simulink model "correlator_2bit_bee2_x2"  (Simulink 7.1)
settings: Solver=ode45  StartTime=0.0  StopTime=2048*5  RelTol=1e-3
StartFcn:
  | warning off Simulink:SL_LoadMdlParameterizedLink;
  | warning off Simulink:SL_OutputNotConnected;
  | warning off Simulink:SL_UsingDefaultMaxStepSize;
undefined symbols (not defined in the file or in its folder): BEE2_usr
libraries: xbsIndex_r4 x1264, cfa_library x320, xps_library x10, casper_library_misc x2
(part 1 of 15)
settings Model: Creator=rprimian  LastModifiedBy=Jonathan Weintroub
settings OptimizationCC: InitFltsAndDblsToZero=off

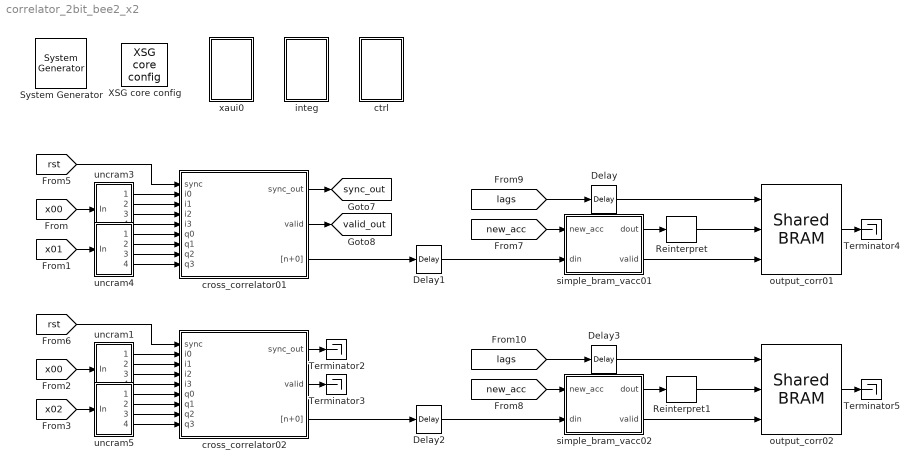
== system correlator_2bit_bee2_x2 ==
  " System Generator" : xbsIndex_r4/ System Generator  Tag=genX  SystemSampleTime=-1  xilinxfamily=virtex2p  part=xc2vp70  speed=-7  package=ff1704  synthesis_tool=XST  clock_wrapper=Clock Enables  directory=./correlator_2bit_bee2_x2/sysgen  testbench=off  simulink_period=1  sysclk_period=3.9063  dcm_input_clock_period=100  incr_netlist=off  trim_vbits=Everywhere in SubSystem  dbl_ovrd=According to Block Masks  core_generation=According to Block Masks  run_coregen=off  deprecated_control=off  eval_field=0  block_type=sysgen
      settings: block_version=11.3
  Delay : xbsIndex_r4/Delay  en=off  latency=2  dbl_ovrd=off  reg_retiming=off  block_type=delay
      settings: block_version=11.4
  Delay1 : xbsIndex_r4/Delay  en=off  latency=4  dbl_ovrd=off  reg_retiming=off  block_type=delay
      settings: block_version=11.4
  Delay2 : xbsIndex_r4/Delay  en=off  latency=4  dbl_ovrd=off  reg_retiming=off  block_type=delay
      settings: block_version=11.4
  Delay3 : xbsIndex_r4/Delay  en=off  latency=2  dbl_ovrd=off  reg_retiming=off  block_type=delay
      settings: block_version=11.4
  Reinterpret : xbsIndex_r4/Reinterpret  force_arith_type=on  arith_type=Unsigned  force_bin_pt=on  bin_pt=0  block_type=reinterpret
      settings: infoedit="Changes signal type without altering the binary representation.   You can changed the signal between signed and unsigned, and relocate the binary point.<br><br>Hardware notes: In hardware this block costs nothing.<br><br>Example:  Suppose the input is 6 bits wide, signed, with 2 fractional bits, and the output is forced to unsigned with 0 fractional bits.  Then an input of -2.0 (1110.00 in binary 2's complement) becomes an output of 56 (111000 in binary)."
  Reinterpret1 : xbsIndex_r4/Reinterpret  force_arith_type=on  arith_type=Unsigned  force_bin_pt=on  bin_pt=0  block_type=reinterpret
      settings: infoedit="Changes signal type without altering the binary representation.   You can changed the signal between signed and unsigned, and relocate the binary point.<br><br>Hardware notes: In hardware this block costs nothing.<br><br>Example:  Suppose the input is 6 bits wide, signed, with 2 fractional bits, and the output is forced to unsigned with 0 fractional bits.  Then an input of -2.0 (1110.00 in binary 2's complement) becomes an output of 56 (111000 in binary)."
  Terminator{2..5} (4 alike) : Terminator
  XSG core config : xps_library/XSG core config  Tag=xps:xsg  SystemSampleTime=-1  hw_sys=BEE2_usr  ibob_linux=off  clk_src=usr_clk2x  iBOB_clk_src=adc0_clk  BEE2_ctrl_clk_src=sys_clk  BEE2_usr_clk_src=usr_clk2x  ROACH_clk_src=adc0_clk  CORR_clk_src=sys_clk  gpio_clk_io_group=iBOB:sma  gpio_clk_bit_index=0  clk_rate=256  sample_period=1  synthesis_tool=XST  mpc_type=powerpc405
  cross_correlator01 : Subsystem  AncestorBlock=cfa_library/cross_correlator  mask(cross_correlator){total_lags=5, n_inputs=2, use_2bit=on, input_bit_width=8, input_bin_pt=7, specify_data_type=on, output_bit_width=12, output_bin_pt=0, quantization=Truncate, overflow=Wrap, specify_mult=off, mult_spec=Behavioral, specify_add=off, add_spec=Fabric, mult_delay=3, add_delay=2, single_output=on}
      mask prompts: total_lags "Total Lags Centered at Zero: (2^?)"; n_inputs "Number of Simultaneous Inputs: (2^?)"; use_2bit "Use special 2-bit binary encoding"; input_bit_width "Input Bit Width:"; input_bin_pt "Input Binary Point:"; specify_data_type "Specify output data type"; output_bit_width "Output Bit Width:"; output_bin_pt "Output Binary Point:"; quantization "Quantization Behavior:"; overflow "Overflow Behavior"; specify_mult "Specify multiplier use (behavioural HDL if not specified)"; mult_spec "Multiplier specification:"; specify_add "Specify adder use (behavioural HDL if not specified)"; add_spec "Adder specification:"; mult_delay "Mult Latency:"; add_delay "Add Latency:"; single_output "Provide single output stream"
      mask help: This time-domain cross correlator accepts two real time-series and outputs the cross-correlation function for the given set of lags. The output is accumulated so that for every two input samples there is exactly one output sample.
      mask init:
      | cross_correlator_init(gcb,...
      |     'total_lags', total_lags,...
      |     'n_inputs', n_inputs,...
      |     'use_2bit', use_2bit,...
      |     'input_bit_width', input_bit_width,...
      |     'input_bin_pt', input_bin_pt,...
      |     'specify_data_type', specify_data_type,...
      |     'output_bit_width', output_bit_width,...
      |     'output_bin_pt', output_bin_pt,...
      |     'quantization', quantization,...
      |     'overflow', overflow,...
      |     'specify_mult', specify_mult,...
      |     'mult_spec', mult_spec,...
      |     'specify_add', specify_add,...
      |     'add_spec', add_spec,...
      |     'mult_delay', mult_delay,...
      |     'add_delay', add_delay,...
      |     'single_output', single_output);
      settings: AttributesFormatString=|
      | 32 lags, Demux: 4, Outputs: 1
      | Special 2-bit binary encoding
      |  Mult: Behavioral, Add: Fabric
      mask StyleString: edit,edit,checkbox,edit,edit,checkbox,edit,edit,popup(Truncate|Round (unbiased: +/- Inf)|Round (unbiased: Even Values)),popup(Wrap|Saturate|Flag as error),checkbox,popup(Behavioral|Core|Embedded),checkbox,popup(Fabric|DSP48),edit,edit,checkbox
      mask CallbackString:
      | ||use_2bit = get_param(gcb,'use_2bit');
      | enables = get_param(gcb, 'MaskEnables');
      | if( strcmp(use_2bit,'on')),
      |     enables{4} = 'off';
      |     enables{5} = 'off';
      | else
      |     enables{4} = 'on';
      |     enables{5} = 'on';
      | end
      | set_param(gcb, 'MaskEnables', enables);
      | |||specify_data_type = get_param(gcb,'specify_data_type');
      | enables = get_param(gcb, 'MaskEnables');
      | if( strcmp(specify_data_type,'off')),
      |     enables{7} = 'off';
      |     enables{8} = 'off';
      |     enables{9} = 'off';
      |     enables{10} = 'off';
      | else
      |     enables{7} = 'on';
      |     enables{8} = 'on';
      |     enables{9} = 'on';
      |     enables{10} = 'on';
      | end
      | set_param(gcb, 'MaskEnables', enables);
      | |||||specify_mult = get_param(gcb,'specify_mult');
      | enables = get_param(gcb, 'MaskEnables');
      | if( strcmp(specify_mult,'off')),
      |     enables{12} = 'off';
      | else
      |     enables{12} = 'on';
      | end
      | set_param(gcb, 'MaskEnables', enables);
      | ||specify_add = get_param(gcb,'specify_add');
      | enables = get_param(gcb, 'MaskEnables');
      | if( strcmp(specify_add,'off')),
      |     enables{14} = 'off';
      | else
      |     enables{14} = 'on';
      | end
      | set_param(gcb, 'MaskEnables', enables);
      | ||||
      mask TabNameString: Basic,Basic,Input,Input,Input,Output,Output,Output,Output,Output,Implementation,Implementation,Implementation,Implementation,Implementation,Implementation,Basic
  cross_correlator02 : Subsystem  AncestorBlock=cfa_library/cross_correlator  mask(cross_correlator){total_lags=5, n_inputs=2, use_2bit=on, input_bit_width=8, input_bin_pt=7, specify_data_type=on, output_bit_width=12, output_bin_pt=0, quantization=Truncate, overflow=Wrap, specify_mult=off, mult_spec=Behavioral, specify_add=off, add_spec=Fabric, mult_delay=3, add_delay=2, single_output=on}
      mask prompts: total_lags "Total Lags Centered at Zero: (2^?)"; n_inputs "Number of Simultaneous Inputs: (2^?)"; use_2bit "Use special 2-bit binary encoding"; input_bit_width "Input Bit Width:"; input_bin_pt "Input Binary Point:"; specify_data_type "Specify output data type"; output_bit_width "Output Bit Width:"; output_bin_pt "Output Binary Point:"; quantization "Quantization Behavior:"; overflow "Overflow Behavior"; specify_mult "Specify multiplier use (behavioural HDL if not specified)"; mult_spec "Multiplier specification:"; specify_add "Specify adder use (behavioural HDL if not specified)"; add_spec "Adder specification:"; mult_delay "Mult Latency:"; add_delay "Add Latency:"; single_output "Provide single output stream"
      mask help: This time-domain cross correlator accepts two real time-series and outputs the cross-correlation function for the given set of lags. The output is accumulated so that for every two input samples there is exactly one output sample.
      mask init: same as correlator_2bit_bee2_x2/cross_correlator01
      settings: AttributesFormatString=|
      | 32 lags, Demux: 4, Outputs: 1
      | Special 2-bit binary encoding
      |  Mult: Behavioral, Add: Fabric
      mask StyleString: edit,edit,checkbox,edit,edit,checkbox,edit,edit,popup(Truncate|Round (unbiased: +/- Inf)|Round (unbiased: Even Values)),popup(Wrap|Saturate|Flag as error),checkbox,popup(Behavioral|Core|Embedded),checkbox,popup(Fabric|DSP48),edit,edit,checkbox
      mask CallbackString:
      | ||use_2bit = get_param(gcb,'use_2bit');
      | enables = get_param(gcb, 'MaskEnables');
      | if( strcmp(use_2bit,'on')),
      |     enables{4} = 'off';
      |     enables{5} = 'off';
      | else
      |     enables{4} = 'on';
      |     enables{5} = 'on';
      | end
      | set_param(gcb, 'MaskEnables', enables);
      | |||specify_data_type = get_param(gcb,'specify_data_type');
      | enables = get_param(gcb, 'MaskEnables');
      | if( strcmp(specify_data_type,'off')),
      |     enables{7} = 'off';
      |     enables{8} = 'off';
      |     enables{9} = 'off';
      |     enables{10} = 'off';
      | else
      |     enables{7} = 'on';
      |     enables{8} = 'on';
      |     enables{9} = 'on';
      |     enables{10} = 'on';
      | end
      | set_param(gcb, 'MaskEnables', enables);
      | |||||specify_mult = get_param(gcb,'specify_mult');
      | enables = get_param(gcb, 'MaskEnables');
      | if( strcmp(specify_mult,'off')),
      |     enables{12} = 'off';
      | else
      |     enables{12} = 'on';
      | end
      | set_param(gcb, 'MaskEnables', enables);
      | ||specify_add = get_param(gcb,'specify_add');
      | enables = get_param(gcb, 'MaskEnables');
      | if( strcmp(specify_add,'off')),
      |     enables{14} = 'off';
      | else
      |     enables{14} = 'on';
      | end
      | set_param(gcb, 'MaskEnables', enables);
      | ||||
      mask TabNameString: Basic,Basic,Input,Input,Input,Output,Output,Output,Output,Output,Implementation,Implementation,Implementation,Implementation,Implementation,Implementation,Basic
  ctrl : Subsystem
      mask Display: fprintf('CTRL');
  integ : Subsystem
      mask Display: fprintf('REG');
  output_corr01 : xps_library/Shared BRAM  Tag=xps:bram  SystemSampleTime=-1  arith_type=Unsigned  addr_width=11  data_width=32  data_bin_pt=0  init_vals=0  sample_rate=1
      link override convert_din: arith_type=Unsigned
      link override correlator_2bit_bee2_output_corr0_data_out: arith_type=Unsigned
      link override correlator_2bit_bee2_x2_output_corr01_data_out: arith_type=Unsigned
      link override correlator_2bit_bee2_x2_output_corr0_data_out: arith_type=Unsigned
      link override correlator_2bit_ibob_output_corr0_data_out: arith_type=Unsigned
      link override cross_correlator_test_corr_out_data_out: arith_type="Signed  (2's comp)"
      link override cross_correlator_test_input_data1_data_out: arith_type=Unsigned
      link override mem/sim_data_in: arith_type=Unsigned
  output_corr02 : xps_library/Shared BRAM  Tag=xps:bram  SystemSampleTime=-1  arith_type=Unsigned  addr_width=11  data_width=32  data_bin_pt=0  init_vals=0  sample_rate=1
      link override convert_din: arith_type=Unsigned
      link override correlator_2bit_bee2_output_corr0_data_out: arith_type=Unsigned
      link override correlator_2bit_bee2_x2_output_corr02_data_out: arith_type=Unsigned
      link override correlator_2bit_bee2_x2_output_corr1_data_out: arith_type=Unsigned
      link override correlator_2bit_ibob_output_corr0_data_out: arith_type=Unsigned
      link override cross_correlator_test_corr_out_data_out: arith_type="Signed  (2's comp)"
      link override cross_correlator_test_input_data1_data_out: arith_type=Unsigned
      link override mem/sim_data_in: arith_type=Unsigned
  simple_bram_vacc01 : Subsystem  mask(simple_bram_vacc){vec_len=32, arith_type=Signed, n_bits=12+5, bin_pt=0, add_latency=2}
      mask prompts: vec_len "Vector length:"; arith_type "Output type:"; n_bits "Bit width (output):"; bin_pt "Binary point (output):"; add_latency "Adder latency:"
      mask help: This simple vector accumulator is based on a shift register. It outputs its previous accumulated vector after a new_acc pulse is received.
      mask init:
      | simple_bram_vacc_init(gcb, ...
      |     'vec_len', vec_len, ...
      |     'arith_type', arith_type, ...
      |     'n_bits', n_bits, ...
      |     'bin_pt', bin_pt);
      mask StyleString: edit,popup(Signed|Unsigned),edit,edit,edit
  simple_bram_vacc02 : Subsystem  mask(simple_bram_vacc){vec_len=32, arith_type=Signed, n_bits=12+5, bin_pt=0, add_latency=2}
      mask prompts: vec_len "Vector length:"; arith_type "Output type:"; n_bits "Bit width (output):"; bin_pt "Binary point (output):"; add_latency "Adder latency:"
      mask help: This simple vector accumulator is based on a shift register. It outputs its previous accumulated vector after a new_acc pulse is received.
      mask init:
      | simple_bram_vacc_init(gcb, ...
      |     'vec_len', vec_len, ...
      |     'arith_type', arith_type, ...
      |     'n_bits', n_bits, ...
      |     'bin_pt', bin_pt);
      mask StyleString: edit,popup(Signed|Unsigned),edit,edit,edit
  uncram1 : Subsystem  AncestorBlock=gavrt_library/uncram  mask(uncram){num_slice=4, slice_width=2, bin_pt=0, arith_type=0}
      mask prompts: num_slice "Number of slices"; slice_width "Slice Width"; bin_pt "Output Binary Point"; arith_type "Output Arithmetic Type (0=Unsigned, 1=Signed)"
      mask help: Takes a concatenated input and slices it up into even pieces and reinterprets them as signed fixed point numbers with a given binary point.
      mask init:
      | uncram_init(gcb, ...
      |     'num_slice', num_slice, ...
      |     'slice_width', slice_width, ...
      |     'bin_pt', bin_pt, ...
      |     'arith_type', arith_type);
      settings: AttributesFormatString=UFix_2_0
  uncram3 : Subsystem  AncestorBlock=gavrt_library/uncram  mask(uncram){num_slice=4, slice_width=2, bin_pt=0, arith_type=0}
      mask prompts: num_slice "Number of slices"; slice_width "Slice Width"; bin_pt "Output Binary Point"; arith_type "Output Arithmetic Type (0=Unsigned, 1=Signed)"
      mask help: Takes a concatenated input and slices it up into even pieces and reinterprets them as signed fixed point numbers with a given binary point.
      mask init:
      | uncram_init(gcb, ...
      |     'num_slice', num_slice, ...
      |     'slice_width', slice_width, ...
      |     'bin_pt', bin_pt, ...
      |     'arith_type', arith_type);
      settings: AttributesFormatString=UFix_2_0
  uncram4 : Subsystem  AncestorBlock=gavrt_library/uncram  mask(uncram){num_slice=4, slice_width=2, bin_pt=0, arith_type=0}
      mask prompts: num_slice "Number of slices"; slice_width "Slice Width"; bin_pt "Output Binary Point"; arith_type "Output Arithmetic Type (0=Unsigned, 1=Signed)"
      mask help: Takes a concatenated input and slices it up into even pieces and reinterprets them as signed fixed point numbers with a given binary point.
      mask init:
      | uncram_init(gcb, ...
      |     'num_slice', num_slice, ...
      |     'slice_width', slice_width, ...
      |     'bin_pt', bin_pt, ...
      |     'arith_type', arith_type);
      settings: AttributesFormatString=UFix_2_0
  uncram5 : Subsystem  AncestorBlock=gavrt_library/uncram  mask(uncram){num_slice=4, slice_width=2, bin_pt=0, arith_type=0}
      mask prompts: num_slice "Number of slices"; slice_width "Slice Width"; bin_pt "Output Binary Point"; arith_type "Output Arithmetic Type (0=Unsigned, 1=Signed)"
      mask help: Takes a concatenated input and slices it up into even pieces and reinterprets them as signed fixed point numbers with a given binary point.
      mask init:
      | uncram_init(gcb, ...
      |     'num_slice', num_slice, ...
      |     'slice_width', slice_width, ...
      |     'bin_pt', bin_pt, ...
      |     'arith_type', arith_type);
      settings: AttributesFormatString=UFix_2_0
  xaui0 : Subsystem
      mask Display: fprintf('XAUI')
  tag scope: sync_out=global, valid_out=global
  wires:
  cross_correlator01/3("[n+0]") -> Delay1/1
  simple_bram_vacc01/1(dout) -> Reinterpret/1
  Reinterpret/1 -> output_corr01/2
  simple_bram_vacc01/2(valid) -> output_corr01/3
  uncram3/1(Out1) -> cross_correlator01/2(i0)
  uncram3/2(Out2) -> cross_correlator01/3(i1)
  uncram3/3(Out3) -> cross_correlator01/4(i2)
  uncram3/4(Out4) -> cross_correlator01/5(i3)
  uncram4/1(Out1) -> cross_correlator01/6(q0)
  uncram4/2(Out2) -> cross_correlator01/7(q1)
  uncram4/3(Out3) -> cross_correlator01/8(q2)
  uncram4/4(Out4) -> cross_correlator01/9(q3)
  output_corr01/1 -> Terminator4/1
  uncram1/1(Out1) -> cross_correlator02/2(i0)
  uncram1/2(Out2) -> cross_correlator02/3(i1)
  uncram1/3(Out3) -> cross_correlator02/4(i2)
  uncram1/4(Out4) -> cross_correlator02/5(i3)
  uncram5/1(Out1) -> cross_correlator02/6(q0)
  uncram5/2(Out2) -> cross_correlator02/7(q1)
  uncram5/3(Out3) -> cross_correlator02/8(q2)
  uncram5/4(Out4) -> cross_correlator02/9(q3)
  cross_correlator02/1(sync_out) -> Terminator2/1
  cross_correlator02/2(valid) -> Terminator3/1
  cross_correlator02/3("[n+0]") -> Delay2/1
  Reinterpret1/1 -> output_corr02/2
  output_corr02/1 -> Terminator5/1
  simple_bram_vacc02/1(dout) -> Reinterpret1/1
  simple_bram_vacc02/2(valid) -> output_corr02/3
  [x00] -> uncram3/1(In)
  [x01] -> uncram4/1(In)
  [x00] -> uncram1/1(In)
  [x02] -> uncram5/1(In)
  [rst] -> cross_correlator01/1(sync)
  [rst] -> cross_correlator02/1(sync)
  [new_acc] -> simple_bram_vacc01/1(new_acc)
  [new_acc] -> simple_bram_vacc02/1(new_acc)
  Delay/1 -> output_corr01/1
  [lags] -> Delay/1
  Delay3/1 -> output_corr02/1
  [lags] -> Delay3/1
  Delay1/1 -> simple_bram_vacc01/2(din)
  Delay2/1 -> simple_bram_vacc02/2(din)
  cross_correlator01/1(sync_out) -> [sync_out]
  cross_correlator01/2(valid) -> [valid_out]
